simulink model "pfb_fft_vacc_float_fft"  (R2016b)
settings: Solver=VariableStepAuto  StartTime=0.0  StopTime=1024*2^5  RelTol=1e-3
undefined symbols (not defined in the file): fft_stages
libraries: xbsIndex_r4 x17526, casper_library_misc x189, casper_library_delays x15, casper_library_sources x4, xbsReplacements_r4 x3, xps_library x1
(part 51 of 140)

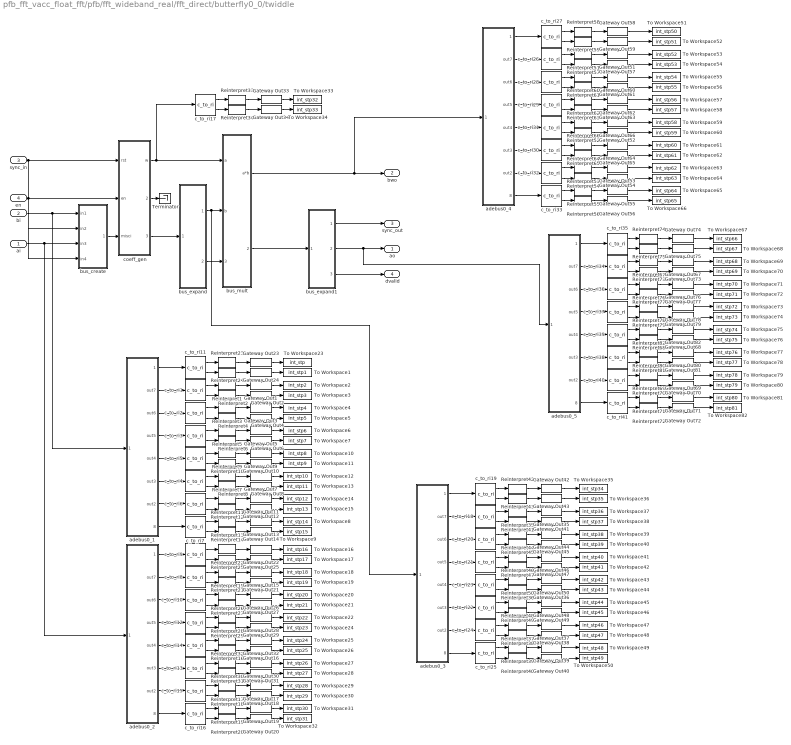
== system pfb_fft_vacc_float_fft/pfb/fft_wideband_real/fft_direct/butterfly0_0/twiddle ==
  ai : Inport
  bi : Inport  Port=2
  sync_in : Inport  Port=3
  en : Inport  Port=4
  Gateway Out{1..82} (82 alike) : xbsIndex_r4/Gateway Out  inherit_from_input=off  hdl_port=on  rows=1  columns=1  UseAsDAC=off  DACChannel='1'  interface=None  automatic_assignment=on  address_offset=hex2dec('00')  intf_name=''  intf_description=''  timing_constraint=None  locs_specified=off  LOCs={}  io_standard={}  block_type=gatewayout
  Reinterpret{1..82} (82 alike) : xbsIndex_r4/Reinterpret  force_arith_type=on  arith_type=Floating-point  force_bin_pt=on  bin_pt=24  block_type=reinterpret
  Terminator : Terminator
  To Workspace1 : ToWorkspace  VariableName=int_stp1  SaveFormat=Timeseries  SampleTime=-1
  To Workspace10 : ToWorkspace  VariableName=int_stp8  SaveFormat=Timeseries  SampleTime=-1
  To Workspace11 : ToWorkspace  VariableName=int_stp9  SaveFormat=Timeseries  SampleTime=-1
  To Workspace12 : ToWorkspace  VariableName=int_stp10  SaveFormat=Timeseries  SampleTime=-1
  To Workspace13 : ToWorkspace  VariableName=int_stp11  SaveFormat=Timeseries  SampleTime=-1
  To Workspace14 : ToWorkspace  VariableName=int_stp12  SaveFormat=Timeseries  SampleTime=-1
  To Workspace15 : ToWorkspace  VariableName=int_stp13  SaveFormat=Timeseries  SampleTime=-1
  To Workspace16 : ToWorkspace  VariableName=int_stp16  SaveFormat=Timeseries  SampleTime=-1
  To Workspace17 : ToWorkspace  VariableName=int_stp17  SaveFormat=Timeseries  SampleTime=-1
  To Workspace18 : ToWorkspace  VariableName=int_stp18  SaveFormat=Timeseries  SampleTime=-1
  To Workspace19 : ToWorkspace  VariableName=int_stp19  SaveFormat=Timeseries  SampleTime=-1
  To Workspace2 : ToWorkspace  VariableName=int_stp2  SaveFormat=Timeseries  SampleTime=-1
  To Workspace20 : ToWorkspace  VariableName=int_stp20  SaveFormat=Timeseries  SampleTime=-1
  To Workspace21 : ToWorkspace  VariableName=int_stp21  SaveFormat=Timeseries  SampleTime=-1
  To Workspace22 : ToWorkspace  VariableName=int_stp22  SaveFormat=Timeseries  SampleTime=-1
  To Workspace23 : ToWorkspace  VariableName=int_stp  SaveFormat=Timeseries  SampleTime=-1
  To Workspace24 : ToWorkspace  VariableName=int_stp23  SaveFormat=Timeseries  SampleTime=-1
  To Workspace25 : ToWorkspace  VariableName=int_stp24  SaveFormat=Timeseries  SampleTime=-1
  To Workspace26 : ToWorkspace  VariableName=int_stp25  SaveFormat=Timeseries  SampleTime=-1
  To Workspace27 : ToWorkspace  VariableName=int_stp26  SaveFormat=Timeseries  SampleTime=-1
  To Workspace28 : ToWorkspace  VariableName=int_stp27  SaveFormat=Timeseries  SampleTime=-1
  To Workspace29 : ToWorkspace  VariableName=int_stp28  SaveFormat=Timeseries  SampleTime=-1
  To Workspace3 : ToWorkspace  VariableName=int_stp3  SaveFormat=Timeseries  SampleTime=-1
  To Workspace30 : ToWorkspace  VariableName=int_stp29  SaveFormat=Timeseries  SampleTime=-1
  To Workspace31 : ToWorkspace  VariableName=int_stp30  SaveFormat=Timeseries  SampleTime=-1
  To Workspace32 : ToWorkspace  VariableName=int_stp31  SaveFormat=Timeseries  SampleTime=-1
  To Workspace33 : ToWorkspace  VariableName=int_stp32  SaveFormat=Timeseries  SampleTime=-1
  To Workspace34 : ToWorkspace  VariableName=int_stp33  SaveFormat=Timeseries  SampleTime=-1
  To Workspace35 : ToWorkspace  VariableName=int_stp34  SaveFormat=Timeseries  SampleTime=-1
  To Workspace36 : ToWorkspace  VariableName=int_stp35  SaveFormat=Timeseries  SampleTime=-1
  To Workspace37 : ToWorkspace  VariableName=int_stp36  SaveFormat=Timeseries  SampleTime=-1
  To Workspace38 : ToWorkspace  VariableName=int_stp37  SaveFormat=Timeseries  SampleTime=-1
  To Workspace39 : ToWorkspace  VariableName=int_stp38  SaveFormat=Timeseries  SampleTime=-1
  To Workspace4 : ToWorkspace  VariableName=int_stp4  SaveFormat=Timeseries  SampleTime=-1
  To Workspace40 : ToWorkspace  VariableName=int_stp39  SaveFormat=Timeseries  SampleTime=-1
  To Workspace41 : ToWorkspace  VariableName=int_stp40  SaveFormat=Timeseries  SampleTime=-1
  To Workspace42 : ToWorkspace  VariableName=int_stp41  SaveFormat=Timeseries  SampleTime=-1
  To Workspace43 : ToWorkspace  VariableName=int_stp42  SaveFormat=Timeseries  SampleTime=-1
  To Workspace44 : ToWorkspace  VariableName=int_stp43  SaveFormat=Timeseries  SampleTime=-1
  To Workspace45 : ToWorkspace  VariableName=int_stp44  SaveFormat=Timeseries  SampleTime=-1
  To Workspace46 : ToWorkspace  VariableName=int_stp45  SaveFormat=Timeseries  SampleTime=-1
  To Workspace47 : ToWorkspace  VariableName=int_stp46  SaveFormat=Timeseries  SampleTime=-1
  To Workspace48 : ToWorkspace  VariableName=int_stp47  SaveFormat=Timeseries  SampleTime=-1
  To Workspace49 : ToWorkspace  VariableName=int_stp48  SaveFormat=Timeseries  SampleTime=-1
  To Workspace5 : ToWorkspace  VariableName=int_stp5  SaveFormat=Timeseries  SampleTime=-1
  To Workspace50 : ToWorkspace  VariableName=int_stp49  SaveFormat=Timeseries  SampleTime=-1
  To Workspace51 : ToWorkspace  VariableName=int_stp50  SaveFormat=Timeseries  SampleTime=-1
  To Workspace52 : ToWorkspace  VariableName=int_stp51  SaveFormat=Timeseries  SampleTime=-1
  To Workspace53 : ToWorkspace  VariableName=int_stp52  SaveFormat=Timeseries  SampleTime=-1
  To Workspace54 : ToWorkspace  VariableName=int_stp53  SaveFormat=Timeseries  SampleTime=-1
  To Workspace55 : ToWorkspace  VariableName=int_stp54  SaveFormat=Timeseries  SampleTime=-1
  To Workspace56 : ToWorkspace  VariableName=int_stp55  SaveFormat=Timeseries  SampleTime=-1
  To Workspace57 : ToWorkspace  VariableName=int_stp56  SaveFormat=Timeseries  SampleTime=-1
  To Workspace58 : ToWorkspace  VariableName=int_stp57  SaveFormat=Timeseries  SampleTime=-1
  To Workspace59 : ToWorkspace  VariableName=int_stp58  SaveFormat=Timeseries  SampleTime=-1
  To Workspace6 : ToWorkspace  VariableName=int_stp6  SaveFormat=Timeseries  SampleTime=-1
  To Workspace60 : ToWorkspace  VariableName=int_stp59  SaveFormat=Timeseries  SampleTime=-1
  To Workspace61 : ToWorkspace  VariableName=int_stp60  SaveFormat=Timeseries  SampleTime=-1
  To Workspace62 : ToWorkspace  VariableName=int_stp61  SaveFormat=Timeseries  SampleTime=-1
  To Workspace63 : ToWorkspace  VariableName=int_stp62  SaveFormat=Timeseries  SampleTime=-1
  To Workspace64 : ToWorkspace  VariableName=int_stp63  SaveFormat=Timeseries  SampleTime=-1
  To Workspace65 : ToWorkspace  VariableName=int_stp64  SaveFormat=Timeseries  SampleTime=-1
  To Workspace66 : ToWorkspace  VariableName=int_stp65  SaveFormat=Timeseries  SampleTime=-1
  To Workspace67 : ToWorkspace  VariableName=int_stp66  SaveFormat=Timeseries  SampleTime=-1
  To Workspace68 : ToWorkspace  VariableName=int_stp67  SaveFormat=Timeseries  SampleTime=-1
  To Workspace69 : ToWorkspace  VariableName=int_stp68  SaveFormat=Timeseries  SampleTime=-1
  To Workspace7 : ToWorkspace  VariableName=int_stp7  SaveFormat=Timeseries  SampleTime=-1
  To Workspace70 : ToWorkspace  VariableName=int_stp69  SaveFormat=Timeseries  SampleTime=-1
  To Workspace71 : ToWorkspace  VariableName=int_stp70  SaveFormat=Timeseries  SampleTime=-1
  To Workspace72 : ToWorkspace  VariableName=int_stp71  SaveFormat=Timeseries  SampleTime=-1
  To Workspace73 : ToWorkspace  VariableName=int_stp72  SaveFormat=Timeseries  SampleTime=-1
  To Workspace74 : ToWorkspace  VariableName=int_stp73  SaveFormat=Timeseries  SampleTime=-1
  To Workspace75 : ToWorkspace  VariableName=int_stp74  SaveFormat=Timeseries  SampleTime=-1
  To Workspace76 : ToWorkspace  VariableName=int_stp75  SaveFormat=Timeseries  SampleTime=-1
  To Workspace77 : ToWorkspace  VariableName=int_stp76  SaveFormat=Timeseries  SampleTime=-1
  To Workspace78 : ToWorkspace  VariableName=int_stp77  SaveFormat=Timeseries  SampleTime=-1
  To Workspace79 : ToWorkspace  VariableName=int_stp78  SaveFormat=Timeseries  SampleTime=-1
  To Workspace8 : ToWorkspace  VariableName=int_stp14  SaveFormat=Timeseries  SampleTime=-1
  To Workspace80 : ToWorkspace  VariableName=int_stp79  SaveFormat=Timeseries  SampleTime=-1
  To Workspace81 : ToWorkspace  VariableName=int_stp80  SaveFormat=Timeseries  SampleTime=-1
  To Workspace82 : ToWorkspace  VariableName=int_stp81  SaveFormat=Timeseries  SampleTime=-1
  To Workspace9 : ToWorkspace  VariableName=int_stp15  SaveFormat=Timeseries  SampleTime=-1
  adebus0_1 : Subsystem  AncestorBlock=casper_library_flow_control/bus_expand  mask(bus_expand){mode=divisions of equal size, outputNum=8, outputWidth=64, outputBinaryPt=0, outputArithmeticType=0, show_format=off, outputToWorkspace=off, variablePrefix=out, outputToModelAsWell=on}
      mask prompts: outputNum "Number of outputs:"; outputWidth "Output width:"; outputBinaryPt "Output binary point position:"; outputArithmeticType "Output arithmetic type (ufix=0, fix=1, bool=2):"; show_format "Print format string?"; outputToWorkspace "Output to workspace?"; variablePrefix "Variable name prefix:"; outputToModelAsWell "Output to model as well?"
      mask help:
      | Expands a 'bus' made using a bus_create or Xilinx
      | concat block.
      | The input will be divided into the specified number
      | of outputs with the specified arithmetic types.
      | Divisions are made from most significant bit.
      mask init: same as pfb_fft_vacc_float_fft/bus_expand1
      settings: AttributesFormatString=8 outputs
      mask TypeOptions mode: divisions of equal size | divisions of arbitrary size
      mask Callback mode: bus_expand_callback();
      mask Callback outputWidth: bus_expand_callback();
      mask Callback outputToWorkspace: bus_expand_callback();
  adebus0_2 : Subsystem  AncestorBlock=casper_library_flow_control/bus_expand  mask(bus_expand){mode=divisions of equal size, outputNum=8, outputWidth=64, outputBinaryPt=0, outputArithmeticType=0, show_format=off, outputToWorkspace=off, variablePrefix=out, outputToModelAsWell=on}
      mask prompts: outputNum "Number of outputs:"; outputWidth "Output width:"; outputBinaryPt "Output binary point position:"; outputArithmeticType "Output arithmetic type (ufix=0, fix=1, bool=2):"; show_format "Print format string?"; outputToWorkspace "Output to workspace?"; variablePrefix "Variable name prefix:"; outputToModelAsWell "Output to model as well?"
      mask help:
      | Expands a 'bus' made using a bus_create or Xilinx
      | concat block.
      | The input will be divided into the specified number
      | of outputs with the specified arithmetic types.
      | Divisions are made from most significant bit.
      mask init: same as pfb_fft_vacc_float_fft/bus_expand1
      settings: AttributesFormatString=8 outputs
      mask TypeOptions mode: divisions of equal size | divisions of arbitrary size
      mask Callback mode: bus_expand_callback();
      mask Callback outputWidth: bus_expand_callback();
      mask Callback outputToWorkspace: bus_expand_callback();
  adebus0_3 : Subsystem  AncestorBlock=casper_library_flow_control/bus_expand  mask(bus_expand){mode=divisions of equal size, outputNum=8, outputWidth=64, outputBinaryPt=0, outputArithmeticType=0, show_format=off, outputToWorkspace=off, variablePrefix=out, outputToModelAsWell=on}
      mask prompts: outputNum "Number of outputs:"; outputWidth "Output width:"; outputBinaryPt "Output binary point position:"; outputArithmeticType "Output arithmetic type (ufix=0, fix=1, bool=2):"; show_format "Print format string?"; outputToWorkspace "Output to workspace?"; variablePrefix "Variable name prefix:"; outputToModelAsWell "Output to model as well?"
      mask help:
      | Expands a 'bus' made using a bus_create or Xilinx
      | concat block.
      | The input will be divided into the specified number
      | of outputs with the specified arithmetic types.
      | Divisions are made from most significant bit.
      mask init: same as pfb_fft_vacc_float_fft/bus_expand1
      settings: AttributesFormatString=8 outputs
      mask TypeOptions mode: divisions of equal size | divisions of arbitrary size
      mask Callback mode: bus_expand_callback();
      mask Callback outputWidth: bus_expand_callback();
      mask Callback outputToWorkspace: bus_expand_callback();
  adebus0_4 : Subsystem  AncestorBlock=casper_library_flow_control/bus_expand  mask(bus_expand){mode=divisions of equal size, outputNum=8, outputWidth=64, outputBinaryPt=0, outputArithmeticType=0, show_format=off, outputToWorkspace=off, variablePrefix=out, outputToModelAsWell=on}
      mask prompts: outputNum "Number of outputs:"; outputWidth "Output width:"; outputBinaryPt "Output binary point position:"; outputArithmeticType "Output arithmetic type (ufix=0, fix=1, bool=2):"; show_format "Print format string?"; outputToWorkspace "Output to workspace?"; variablePrefix "Variable name prefix:"; outputToModelAsWell "Output to model as well?"
      mask help:
      | Expands a 'bus' made using a bus_create or Xilinx
      | concat block.
      | The input will be divided into the specified number
      | of outputs with the specified arithmetic types.
      | Divisions are made from most significant bit.
      mask init: same as pfb_fft_vacc_float_fft/bus_expand1
      settings: AttributesFormatString=8 outputs
      mask TypeOptions mode: divisions of equal size | divisions of arbitrary size
      mask Callback mode: bus_expand_callback();
      mask Callback outputWidth: bus_expand_callback();
      mask Callback outputToWorkspace: bus_expand_callback();
  adebus0_5 : Subsystem  AncestorBlock=casper_library_flow_control/bus_expand  mask(bus_expand){mode=divisions of equal size, outputNum=8, outputWidth=64, outputBinaryPt=0, outputArithmeticType=0, show_format=off, outputToWorkspace=off, variablePrefix=out, outputToModelAsWell=on}
      mask prompts: outputNum "Number of outputs:"; outputWidth "Output width:"; outputBinaryPt "Output binary point position:"; outputArithmeticType "Output arithmetic type (ufix=0, fix=1, bool=2):"; show_format "Print format string?"; outputToWorkspace "Output to workspace?"; variablePrefix "Variable name prefix:"; outputToModelAsWell "Output to model as well?"
      mask help:
      | Expands a 'bus' made using a bus_create or Xilinx
      | concat block.
      | The input will be divided into the specified number
      | of outputs with the specified arithmetic types.
      | Divisions are made from most significant bit.
      mask init: same as pfb_fft_vacc_float_fft/bus_expand1
      settings: AttributesFormatString=8 outputs
      mask TypeOptions mode: divisions of equal size | divisions of arbitrary size
      mask Callback mode: bus_expand_callback();
      mask Callback outputWidth: bus_expand_callback();
      mask Callback outputToWorkspace: bus_expand_callback();
  bus_create : Subsystem  AncestorBlock=casper_library_flow_control/bus_create  mask(bus_create){inputNum=4}
      mask prompts: inputNum "Number of inputs:"
      mask init:
      | bus_create_init(gcb,...
      |     'inputNum', inputNum);
  bus_expand : Subsystem  AncestorBlock=casper_library_flow_control/bus_expand  mask(bus_expand){mode=divisions of arbitrary size, outputNum=1, outputWidth=[n_inputs*input_bit_width*2, 2+(n_inputs*input_bit_width*2)], outputBinaryPt=[0 0], outputArithmeticType=[0 0], show_format=off, outputToWorkspace=off, variablePrefix=out, outputToModelAsWell=on}
      mask prompts: outputNum "Number of outputs:"; outputWidth "Output width [msb ... lsb]:"; outputBinaryPt "Output binary point position [msb ... lsb]:"; outputArithmeticType "Output arithmetic type (ufix=0, fix=1, bool=2) [msb ... lsb]:"; show_format "Print format string?"; outputToWorkspace "Output to workspace?"; variablePrefix "Variable name prefix:"; outputToModelAsWell "Output to model as well?"
      mask help:
      | Expands a 'bus' made using a bus_create or Xilinx
      | concat block.
      | The input will be divided into the specified number
      | of outputs with the specified arithmetic types.
      | Divisions are made from most significant bit.
      mask init: same as pfb_fft_vacc_float_fft/bus_expand1
      settings: AttributesFormatString=2 outputs
      mask TypeOptions mode: divisions of equal size | divisions of arbitrary size
      mask Callback mode: bus_expand_callback();
      mask Callback outputWidth: bus_expand_callback();
      mask Callback outputToWorkspace: bus_expand_callback();
  bus_expand1 : Subsystem  AncestorBlock=casper_library_flow_control/bus_expand  mask(bus_expand){mode=divisions of arbitrary size, outputNum=1, outputWidth=[1 512 1], outputBinaryPt=[0 0 0], outputArithmeticType=[2 0 2], show_format=off, outputToWorkspace=off, variablePrefix=out, outputToModelAsWell=on}
      mask prompts: outputNum "Number of outputs:"; outputWidth "Output width [msb ... lsb]:"; outputBinaryPt "Output binary point position [msb ... lsb]:"; outputArithmeticType "Output arithmetic type (ufix=0, fix=1, bool=2) [msb ... lsb]:"; show_format "Print format string?"; outputToWorkspace "Output to workspace?"; variablePrefix "Variable name prefix:"; outputToModelAsWell "Output to model as well?"
      mask help:
      | Expands a 'bus' made using a bus_create or Xilinx
      | concat block.
      | The input will be divided into the specified number
      | of outputs with the specified arithmetic types.
      | Divisions are made from most significant bit.
      mask init: same as pfb_fft_vacc_float_fft/bus_expand1
      settings: AttributesFormatString=3 outputs
      mask TypeOptions mode: divisions of equal size | divisions of arbitrary size
      mask Callback mode: bus_expand_callback();
      mask Callback outputWidth: bus_expand_callback();
      mask Callback outputToWorkspace: bus_expand_callback();
  bus_mult : Subsystem  AncestorBlock=casper_library_bus/bus_mult  mask(bus_mult){n_bits_a=coeff_bit_width, bin_pt_a=coeff_bit_width-1, type_a=1, cmplx_a=on, n_bits_b=[32 32 32 32 32 32 32 32], bin_pt_b=bin_pt_in, type_b=1, cmplx_b=on, misc=on, n_bits_out=input_bit_width+coeff_bit_width+1, bin_pt_out=(bin_pt_in+coeff_bit_width-1), type_out=1, quantization=0, overflow=0, mult_latency=mult_latency, add_latency=add_latency, conv_latency=0, max_fanout=max_fanout, fan_latency=4, multiplier_implementation=behavioral HDL}
      mask prompts: n_bits_a "a input bit widths"; bin_pt_a "a input binary points"; type_a "a input type (Unsigned=0, Signed=1)"; cmplx_a "a input complex"; n_bits_b "b input bit widths"; bin_pt_b "b input binary points"; type_b "b input type (Unsigned=0, Signed=1)"; cmplx_b "b input complex"; misc "misc support"; n_bits_out "output bit widths"; bin_pt_out "output binary points"; type_out "output type (Unsigned=0, Signed=1)"; quantization "quantization strategy (Truncate=0, Round  (unbiased: +/- Inf)=1)"; overflow "overflow strategy (Wrap=0, Saturate=1, Flag as error=2)"; mult_latency "multiplier latency"; add_latency "adder latency"; conv_latency "convert latency"; max_fanout "limit fanout to ?"; fan_latency "fanout register latency"; multiplier_implementation "multiplier implementation"
      mask help: Multiply components of two busses
      mask init: same as pfb_fft_vacc_float_fft/bus_mult
      mask TypeOptions multiplier_implementation: behavioral HDL | standard core | embedded multiplier core
  c_to_ri{1..41} (41 alike) : casper_library_misc/c_to_ri  n_bits=32  bin_pt=0
      settings: AttributesFormatString=32_0 r/i
  coeff_gen : Subsystem  AncestorBlock=casper_library_ffts_twiddle_coeff_gen/coeff_gen  mask(coeff_gen){FFTSize=FFTSize, Coeffs=[0 512 256 768 128 640 384 896 64 576 320 832 192 704 448 960 32 544 288 800 160 672 416 928 96 608 352 864 224 736 480 992 16 528 272 784 144 656 400 912 80 592 336 848 208 720 464 976 48 560 304 816 176 688 432 944 112 624 368 880 240 752 496 1008 8 520 264 776 136 648 392 904 72 584 328 840 200 712 456 968 40 552 296 808 168 680 424 936 104 616 360 872 232 744 488 1000 24 536 280 792 152 664 408 920 88 600 344 856 216 728 472 984 56 568 312 824 184 696 440 952 120 632 376 888 248 760 504 1016 4 516 260 772 132 644 388 900 68 580 324 836 196 708 452 964 36 548 292 804 164 676 420 932 100 612 356 868 228 740 484 996 20 532 276 788 148 660 404 916 84 596 340 852 212 724 468 980 52 564 308 820 180 692 436 948 116 628 372 884 244 756 500 1012 12 524 268 780 140 652 396 908 76 588 332 844 204 716 460 972 44 556 300 812 172 684 428 940 108 620 364 876 236 748 492 1004 28 540 284 796 156 668 412 924 92 604 348 860 220 732 476 988 60 572 316 828 188 700 444 956 124 636 380 892 252 764 508 1020 2 514 258 770 130 642 386 898 66 578 322 834 194 706 450 962 34 546 290 802 162 674 418 930 98 610 354 866 226 738 482 994 18 530 274 786 146 658 402 914 82 594 338 850 210 722 466 978 50 562 306 818 178 690 434 946 114 626 370 882 242 754 498 1010 10 522 266 778 138 650 394 906 74 586 330 842 202 714 458 970 42 554 298 810 170 682 426 938 106 618 362 874 234 746 490 1002 26 538 282 794 154 666 410 922 90 602 346 858 218 730 474 986 58 570 314 826 186 698 442 954 122 634 378 890 250 762 506 1018 6 518 262 774 134 646 390 902 70 582 326 838 198 710 454 966 38 550 294 806 166 678 422 934 102 614 358 870 230 742 486 998 22 534 278 790 150 662 406 918 86 598 342 854 214 726 470 982 54 566 310 822 182 694 438 950 118 630 374 886 246 758 502 1014 14 526 270 782 142 654 398 910 78 590 334 846 206 718 462 974 46 558 302 814 174 686 430 942 110 622 366 878 238 750 494 1006 30 542 286 798 158 670 414 926 94 606 350 862 222 734 478 990 62 574 318 830 190 702 446 958 126 638 382 894 254 766 510 1022 1 513 257 769 129 641 385 897 65 577 321 833 193 705 449 961 33 545 289 801 161 673 417 929 97 609 353 865 225 737 481 993 17 529 273 785 145 657 401 913 81 593 337 849 209 721 465 977 49 561 305 817 177 689 433 945 113 625 369 881 241 753 497 1009 9 521 265 777 137 649 393 905 73 585 329 841 201 713 457 969 41 553 297 809 169 681 425 937 105 617 361 873 233 745 489 1001 25 537 281 793 153 665 409 921 89 601 345 857 217 729 473 985 57 569 313 825 185 697 441 953 121 633 377 889 249 761 505 1017 5 517 261 773 133 645 389 901 69 581 325 837 197 709 453 965 37 549 293 805 165 677 421 933 101 613 357 869 229 741 485 997 21 533 277 789 149 661 405 917 85 597 341 853 213 725 469 981 53 565 309 821 181 693 437 949 117 629 373 885 245 757 501 1013 13 525 269 781 141 653 397 909 77 589 333 845 205 717 461 973 45 557 301 813 173 685 429 941 109 621 365 877 237 749 493 1005 29 541 285 797 157 669 413 925 93 605 349 861 221 733 477 989 61 573 317 829 189 701 445 957 125 637 381 893 253 765 509 1021 3 515 259 771 131 643 387 899 67 579 323 835 195 707 451 963 35 547 291 803 163 675 419 931 99 611 355 867 227 739 483 995 19 531 275 787 147 659 403 915 83 595 339 851 211 723 467 979 51 563 307 819 179 691 435 947 115 627 371 883 243 755 499 1011 11 523 267 779 139 651 395 907 75 587 331 843 203 715 459 971 43 555 299 811 171 683 427 939 107 619 363 875 235 747 491 1003 27 539 283 795 155 667 411 923 91 603 347 859 219 731 475 987 59 571 315 827 187 699 443 955 123 635 379 891 251 763 507 1019 7 519 263 775 135 647 391 903 71 583 327 839 199 711 455 967 39 551 295 807 167 679 423 935 103 615 359 871 231 743 487 999 23 535 279 791 151 663 407 919 87 599 343 855 215 727 471 983 55 567 311 823 183 695 439 951 119 631 375 887 247 759 503 1015 15 527 271 783 143 655 399 911 79 591 335 847 207 719 463 975 47 559 303 815 175 687 431 943 111 623 367 879 239 751 495 1007 31 543 287 799 159 671 415 927 95 607 351 863 223 735 479 991 63 575 319 831 191 703 447 959 127 639 383 895 255 767 511 1023], coeff_bit_width=coeff_bit_width, StepPeriod=StepPeriod, async=on, misc=on, bram_latency=bram_latency, mult_latency=mult_latency, add_latency=add_latency, conv_latency=conv_latency, coeffs_bit_limit=coeffs_bit_limit, coeff_sharing=on, coeff_decimation=on, coeff_generation=on, cal_bits=cal_bits, n_bits_rotation=n_bits_rotation, quantization=Round  (unbiased: +/- Inf)}
      mask prompts: FFTSize "fft size (2^?)"; Coeffs "Coefficients"; coeff_bit_width "Coefficient Bit Width:"; StepPeriod "Step Period: (2^?)"; async "asynchronous operation"; misc "misc port"; bram_latency "BRAM latency"; mult_latency "Multiplier latency"; add_latency "Adder latency"; conv_latency "Convert latency"; coeffs_bit_limit "Number bits above which to store stage's coefficients in BRAM (2^? bits)"; coeff_sharing "BRAM sharing in coeff storage"; coeff_decimation "Store a fraction of coeff factors where useful"; coeff_generation "Generate coeffs with multipliers where useful"; cal_bits "Number calibration locations when generating coeffs (2^?)"; n_bits_rotation "Rotation vector resolution"; quantization "Quantization strategy"
      mask help:
      | Generates a sequence of complex coefficients for use in generating twiddle
      | factors for FFTs.
      mask init:
      | coeff_gen_init(gcb, ...
      |    'FFTSize', FFTSize, ...
      |    'Coeffs', Coeffs, ...
      |    'coeff_bit_width', coeff_bit_width, ...
      |    'StepPeriod', StepPeriod, ...
      |    'async', async, ...
      |    'misc', misc, ...
      |    'bram_latency', bram_latency, ...
      |    'mult_latency', mult_latency, ...
      |    'add_latency', add_latency, ...
      |    'conv_latency', conv_latency, ...
      |    'coeffs_bit_limit', coeffs_bit_limit, ...
      |    'coeff_sharing', coeff_sharing, ...
      |    'coeff_decimation', coeff_decimation, ...
      |    'coeff_generation', coeff_generation, ...
      |    'cal_bits', cal_bits, ...
      |    'n_bits_rotation', n_bits_rotation, ...
      |    'quantization', quantization);
      settings: AttributesFormatString=1024 @ (32,31)
      mask TypeOptions quantization: Truncate | Round  (unbiased: +/- Inf) | Round  (unbiased: Even Values)
  ao : Outport
  bwo : Outport  Port=2
  sync_out : Outport  Port=3
  dvalid : Outport  Port=4
  wires:
  bi/1 -> adebus0_1/1(bus_in), bus_create/1(in1)
  sync_in/1 -> bus_create/2(in2), coeff_gen/1(rst)
  ai/1 -> adebus0_2/1(bus_in), bus_create/3(in3)
  en/1 -> bus_create/4(in4), coeff_gen/2(en)
  bus_create/1(bus_out) -> coeff_gen/3(misci)
  coeff_gen/2(dvalid) -> Terminator/1
  coeff_gen/3(misco) -> bus_expand/1(bus_in)
  coeff_gen/1(w) -> c_to_ri17/1, bus_mult/1(a)
  bus_expand/2(lsb_out1) -> bus_mult/3(misci)
  bus_expand/1(msb_out2) -> adebus0_3/1(bus_in), bus_mult/2(b)
  bus_mult/1(a*b) -> adebus0_4/1(bus_in), bwo/1
  bus_mult/2(misco) -> bus_expand1/1(bus_in)
  bus_expand1/1(msb_out3) -> sync_out/1
  bus_expand1/2(out2) -> adebus0_5/1(bus_in), ao/1
  bus_expand1/3(lsb_out1) -> dvalid/1
  Gateway Out23/1 -> To Workspace23/1
  c_to_ri11/1 -> Reinterpret23/1
  c_to_ri11/2 -> Reinterpret24/1
  Reinterpret23/1 -> Gateway Out23/1
  Reinterpret24/1 -> Gateway Out24/1
  c_to_ri1/1 -> Reinterpret1/1
  c_to_ri1/2 -> Reinterpret2/1
  Reinterpret1/1 -> Gateway Out1/1
  Reinterpret2/1 -> Gateway Out2/1
  c_to_ri2/1 -> Reinterpret3/1
  c_to_ri2/2 -> Reinterpret4/1
  Reinterpret3/1 -> Gateway Out3/1
  Reinterpret4/1 -> Gateway Out4/1
  c_to_ri3/1 -> Reinterpret5/1
  c_to_ri3/2 -> Reinterpret6/1
  Reinterpret5/1 -> Gateway Out5/1
  Reinterpret6/1 -> Gateway Out6/1
  Gateway Out24/1 -> To Workspace1/1
  Gateway Out1/1 -> To Workspace2/1
  Gateway Out2/1 -> To Workspace3/1
  c_to_ri5/1 -> Reinterpret9/1
  c_to_ri5/2 -> Reinterpret10/1
  Reinterpret9/1 -> Gateway Out9/1
  Reinterpret10/1 -> Gateway Out10/1
  c_to_ri4/1 -> Reinterpret7/1
  c_to_ri4/2 -> Reinterpret8/1
  Reinterpret7/1 -> Gateway Out7/1
  Reinterpret8/1 -> Gateway Out8/1
  c_to_ri6/1 -> Reinterpret11/1
  c_to_ri6/2 -> Reinterpret12/1
  Reinterpret11/1 -> Gateway Out11/1
  Reinterpret12/1 -> Gateway Out12/1
  c_to_ri7/1 -> Reinterpret13/1
  c_to_ri7/2 -> Reinterpret14/1
  Reinterpret13/1 -> Gateway Out13/1
  Reinterpret14/1 -> Gateway Out14/1
  adebus0_1/8(lsb_out1) -> c_to_ri7/1
  adebus0_1/7(out2) -> c_to_ri6/1
  adebus0_1/6(out3) -> c_to_ri4/1
  adebus0_1/5(out4) -> c_to_ri5/1
  adebus0_1/4(out5) -> c_to_ri3/1
  adebus0_1/3(out6) -> c_to_ri2/1
  adebus0_1/2(out7) -> c_to_ri1/1
  adebus0_1/1(msb_out8) -> c_to_ri11/1
  c_to_ri9/1 -> Reinterpret22/1
  c_to_ri9/2 -> Reinterpret25/1
  Reinterpret22/1 -> Gateway Out22/1
  Reinterpret25/1 -> Gateway Out25/1
  c_to_ri8/1 -> Reinterpret15/1
  c_to_ri8/2 -> Reinterpret21/1
  Reinterpret15/1 -> Gateway Out15/1
  Reinterpret21/1 -> Gateway Out21/1
  c_to_ri10/1 -> Reinterpret26/1
  c_to_ri10/2 -> Reinterpret27/1
  Reinterpret26/1 -> Gateway Out26/1
  Reinterpret27/1 -> Gateway Out27/1
  c_to_ri12/1 -> Reinterpret28/1
  c_to_ri12/2 -> Reinterpret29/1
  Reinterpret28/1 -> Gateway Out28/1
  Reinterpret29/1 -> Gateway Out29/1
  c_to_ri14/1 -> Reinterpret32/1
  c_to_ri14/2 -> Reinterpret16/1
  Reinterpret32/1 -> Gateway Out32/1
  Reinterpret16/1 -> Gateway Out16/1
  c_to_ri13/1 -> Reinterpret30/1
  c_to_ri13/2 -> Reinterpret31/1
  Reinterpret30/1 -> Gateway Out30/1
  Reinterpret31/1 -> Gateway Out31/1
  c_to_ri15/1 -> Reinterpret17/1
  c_to_ri15/2 -> Reinterpret18/1
  Reinterpret17/1 -> Gateway Out17/1
  Reinterpret18/1 -> Gateway Out18/1
  c_to_ri16/1 -> Reinterpret19/1
  c_to_ri16/2 -> Reinterpret20/1
  Reinterpret19/1 -> Gateway Out19/1
  Reinterpret20/1 -> Gateway Out20/1
  adebus0_2/8(lsb_out1) -> c_to_ri16/1
  adebus0_2/7(out2) -> c_to_ri15/1
  adebus0_2/6(out3) -> c_to_ri13/1
  adebus0_2/5(out4) -> c_to_ri14/1
  adebus0_2/4(out5) -> c_to_ri12/1
  adebus0_2/3(out6) -> c_to_ri10/1
  adebus0_2/2(out7) -> c_to_ri8/1
  adebus0_2/1(msb_out8) -> c_to_ri9/1
  c_to_ri17/1 -> Reinterpret33/1
  c_to_ri17/2 -> Reinterpret34/1
  Reinterpret33/1 -> Gateway Out33/1
  Reinterpret34/1 -> Gateway Out34/1
  c_to_ri19/1 -> Reinterpret42/1
  c_to_ri19/2 -> Reinterpret43/1
  Reinterpret42/1 -> Gateway Out42/1
  Reinterpret43/1 -> Gateway Out43/1
  c_to_ri18/1 -> Reinterpret35/1
  c_to_ri18/2 -> Reinterpret41/1
  Reinterpret35/1 -> Gateway Out35/1
  Reinterpret41/1 -> Gateway Out41/1
  c_to_ri20/1 -> Reinterpret44/1
  c_to_ri20/2 -> Reinterpret45/1
  Reinterpret44/1 -> Gateway Out44/1
  Reinterpret45/1 -> Gateway Out45/1
  c_to_ri21/1 -> Reinterpret46/1
  c_to_ri21/2 -> Reinterpret47/1
  Reinterpret46/1 -> Gateway Out46/1
  Reinterpret47/1 -> Gateway Out47/1
  c_to_ri23/1 -> Reinterpret50/1
  c_to_ri23/2 -> Reinterpret36/1
  Reinterpret50/1 -> Gateway Out50/1
  Reinterpret36/1 -> Gateway Out36/1
  c_to_ri22/1 -> Reinterpret48/1
  c_to_ri22/2 -> Reinterpret49/1
  Reinterpret48/1 -> Gateway Out48/1
  Reinterpret49/1 -> Gateway Out49/1
  c_to_ri24/1 -> Reinterpret37/1
  c_to_ri24/2 -> Reinterpret38/1
  Reinterpret37/1 -> Gateway Out37/1
  Reinterpret38/1 -> Gateway Out38/1
  c_to_ri25/1 -> Reinterpret39/1
  c_to_ri25/2 -> Reinterpret40/1
  Reinterpret39/1 -> Gateway Out39/1
  Reinterpret40/1 -> Gateway Out40/1
  adebus0_3/8(lsb_out1) -> c_to_ri25/1
  adebus0_3/7(out2) -> c_to_ri24/1
  adebus0_3/6(out3) -> c_to_ri22/1
  adebus0_3/5(out4) -> c_to_ri23/1
  adebus0_3/4(out5) -> c_to_ri21/1
  adebus0_3/3(out6) -> c_to_ri20/1
  adebus0_3/2(out7) -> c_to_ri18/1
  adebus0_3/1(msb_out8) -> c_to_ri19/1
  c_to_ri27/1 -> Reinterpret58/1
  c_to_ri27/2 -> Reinterpret59/1
  Reinterpret58/1 -> Gateway Out58/1
  Reinterpret59/1 -> Gateway Out59/1
  c_to_ri26/1 -> Reinterpret51/1
  c_to_ri26/2 -> Reinterpret57/1
  Reinterpret51/1 -> Gateway Out51/1
  Reinterpret57/1 -> Gateway Out57/1
  c_to_ri28/1 -> Reinterpret60/1
  c_to_ri28/2 -> Reinterpret61/1
  Reinterpret60/1 -> Gateway Out60/1
  Reinterpret61/1 -> Gateway Out61/1
  c_to_ri29/1 -> Reinterpret62/1
  c_to_ri29/2 -> Reinterpret63/1
  Reinterpret62/1 -> Gateway Out62/1
  Reinterpret63/1 -> Gateway Out63/1
  c_to_ri31/1 -> Reinterpret66/1
  c_to_ri31/2 -> Reinterpret52/1
  Reinterpret66/1 -> Gateway Out66/1
  Reinterpret52/1 -> Gateway Out52/1
  c_to_ri30/1 -> Reinterpret64/1
  c_to_ri30/2 -> Reinterpret65/1
  Reinterpret64/1 -> Gateway Out64/1
  Reinterpret65/1 -> Gateway Out65/1
  c_to_ri32/1 -> Reinterpret53/1
  c_to_ri32/2 -> Reinterpret54/1
  Reinterpret53/1 -> Gateway Out53/1
  Reinterpret54/1 -> Gateway Out54/1
  c_to_ri33/1 -> Reinterpret55/1
  c_to_ri33/2 -> Reinterpret56/1
  Reinterpret55/1 -> Gateway Out55/1
  Reinterpret56/1 -> Gateway Out56/1
  adebus0_4/8(lsb_out1) -> c_to_ri33/1
  adebus0_4/7(out2) -> c_to_ri32/1
  adebus0_4/6(out3) -> c_to_ri30/1
  adebus0_4/5(out4) -> c_to_ri31/1
  adebus0_4/4(out5) -> c_to_ri29/1
  adebus0_4/3(out6) -> c_to_ri28/1
  adebus0_4/2(out7) -> c_to_ri26/1
  adebus0_4/1(msb_out8) -> c_to_ri27/1
  c_to_ri35/1 -> Reinterpret74/1
  c_to_ri35/2 -> Reinterpret75/1
  Reinterpret74/1 -> Gateway Out74/1
  Reinterpret75/1 -> Gateway Out75/1
  c_to_ri34/1 -> Reinterpret67/1
  c_to_ri34/2 -> Reinterpret73/1
  Reinterpret67/1 -> Gateway Out67/1
  Reinterpret73/1 -> Gateway Out73/1
  c_to_ri36/1 -> Reinterpret76/1
  c_to_ri36/2 -> Reinterpret77/1
  Reinterpret76/1 -> Gateway Out76/1
  Reinterpret77/1 -> Gateway Out77/1
  c_to_ri37/1 -> Reinterpret78/1
  c_to_ri37/2 -> Reinterpret79/1
  Reinterpret78/1 -> Gateway Out78/1
  Reinterpret79/1 -> Gateway Out79/1
  c_to_ri39/1 -> Reinterpret82/1
  c_to_ri39/2 -> Reinterpret68/1
  Reinterpret82/1 -> Gateway Out82/1
  Reinterpret68/1 -> Gateway Out68/1
  c_to_ri38/1 -> Reinterpret80/1
  c_to_ri38/2 -> Reinterpret81/1
  Reinterpret80/1 -> Gateway Out80/1
  Reinterpret81/1 -> Gateway Out81/1
  c_to_ri40/1 -> Reinterpret69/1
  c_to_ri40/2 -> Reinterpret70/1
  Reinterpret69/1 -> Gateway Out69/1
  Reinterpret70/1 -> Gateway Out70/1
  c_to_ri41/1 -> Reinterpret71/1
  c_to_ri41/2 -> Reinterpret72/1
  Reinterpret71/1 -> Gateway Out71/1
  Reinterpret72/1 -> Gateway Out72/1
  adebus0_5/8(lsb_out1) -> c_to_ri41/1
  adebus0_5/7(out2) -> c_to_ri40/1
  adebus0_5/6(out3) -> c_to_ri38/1
  adebus0_5/5(out4) -> c_to_ri39/1
  adebus0_5/4(out5) -> c_to_ri37/1
  adebus0_5/3(out6) -> c_to_ri36/1
  adebus0_5/2(out7) -> c_to_ri34/1
  adebus0_5/1(msb_out8) -> c_to_ri35/1
  Gateway Out3/1 -> To Workspace4/1
  Gateway Out4/1 -> To Workspace5/1
  Gateway Out5/1 -> To Workspace6/1
  Gateway Out6/1 -> To Workspace7/1
  Gateway Out9/1 -> To Workspace10/1
  Gateway Out10/1 -> To Workspace11/1
  Gateway Out7/1 -> To Workspace12/1
  Gateway Out8/1 -> To Workspace13/1
  Gateway Out11/1 -> To Workspace14/1
  Gateway Out12/1 -> To Workspace15/1
  Gateway Out13/1 -> To Workspace8/1
  Gateway Out14/1 -> To Workspace9/1
  Gateway Out22/1 -> To Workspace16/1
  Gateway Out25/1 -> To Workspace17/1
  Gateway Out15/1 -> To Workspace18/1
  Gateway Out21/1 -> To Workspace19/1
  Gateway Out26/1 -> To Workspace20/1
  Gateway Out27/1 -> To Workspace21/1
  Gateway Out28/1 -> To Workspace22/1
  Gateway Out29/1 -> To Workspace24/1
  Gateway Out32/1 -> To Workspace25/1
  Gateway Out16/1 -> To Workspace26/1
  Gateway Out30/1 -> To Workspace27/1
  Gateway Out31/1 -> To Workspace28/1
  Gateway Out17/1 -> To Workspace29/1
  Gateway Out18/1 -> To Workspace30/1
  Gateway Out19/1 -> To Workspace31/1
  Gateway Out20/1 -> To Workspace32/1
  Gateway Out33/1 -> To Workspace33/1
  Gateway Out34/1 -> To Workspace34/1
  Gateway Out42/1 -> To Workspace35/1
  Gateway Out43/1 -> To Workspace36/1
  Gateway Out35/1 -> To Workspace37/1
  Gateway Out41/1 -> To Workspace38/1
  Gateway Out44/1 -> To Workspace39/1
  Gateway Out45/1 -> To Workspace40/1
  Gateway Out46/1 -> To Workspace41/1
  Gateway Out47/1 -> To Workspace42/1
  Gateway Out50/1 -> To Workspace43/1
  Gateway Out36/1 -> To Workspace44/1
  Gateway Out48/1 -> To Workspace45/1
  Gateway Out49/1 -> To Workspace46/1
  Gateway Out37/1 -> To Workspace47/1
  Gateway Out38/1 -> To Workspace48/1
  Gateway Out39/1 -> To Workspace49/1
  Gateway Out40/1 -> To Workspace50/1
  Gateway Out58/1 -> To Workspace51/1
  Gateway Out59/1 -> To Workspace52/1
  Gateway Out51/1 -> To Workspace53/1
  Gateway Out57/1 -> To Workspace54/1
  Gateway Out60/1 -> To Workspace55/1
  Gateway Out61/1 -> To Workspace56/1
  Gateway Out62/1 -> To Workspace57/1
  Gateway Out63/1 -> To Workspace58/1
  Gateway Out66/1 -> To Workspace59/1
  Gateway Out52/1 -> To Workspace60/1
  Gateway Out64/1 -> To Workspace61/1
  Gateway Out65/1 -> To Workspace62/1
  Gateway Out53/1 -> To Workspace63/1
  Gateway Out54/1 -> To Workspace64/1
  Gateway Out55/1 -> To Workspace65/1
  Gateway Out56/1 -> To Workspace66/1
  Gateway Out74/1 -> To Workspace67/1
  Gateway Out75/1 -> To Workspace68/1
  Gateway Out67/1 -> To Workspace69/1
  Gateway Out73/1 -> To Workspace70/1
  Gateway Out76/1 -> To Workspace71/1
  Gateway Out77/1 -> To Workspace72/1
  Gateway Out78/1 -> To Workspace73/1
  Gateway Out79/1 -> To Workspace74/1
  Gateway Out82/1 -> To Workspace75/1
  Gateway Out68/1 -> To Workspace76/1
  Gateway Out80/1 -> To Workspace77/1
  Gateway Out81/1 -> To Workspace78/1
  Gateway Out69/1 -> To Workspace79/1
  Gateway Out70/1 -> To Workspace80/1
  Gateway Out71/1 -> To Workspace81/1
  Gateway Out72/1 -> To Workspace82/1
